simulink library "inp"  (Simulink 8.2)
settings: Solver=ode45  SolverMode=SingleTasking  StartTime=0.0  StopTime=10.0  RelTol=1e-3
undefined symbols (not defined in the file or in its folder): C_TICK_TIME_S, ENUM_FAULTSTATE_FAIL, ENUM_FAULTSTATE_INDETERMINATE, ENUM_FAULTSTATE_PASS, ENUM_KEYSWITCH_ON, FALSE, KINP_ABS1CANCommuOk_flg_ovrdflg, KINP_ABS1CANCommuOk_flg_ovrdval, KINP_ABS1CANPeriodTime_s, KINP_ABS1CANRolCntOk_flg_ovrdflg, KINP_ABS1CANRolCntOk_flg_ovrdval, KINP_ABS2CANChkSumOk_flg_ovrdflg, KINP_ABS2CANChkSumOk_flg_ovrdval, KINP_ABS2CANCommuOk_flg_ovrdflg, KINP_ABS2CANCommuOk_flg_ovrdval, KINP_ABS2CANPeriodTime_s, KINP_ABS2CANRolCntOk_flg_ovrdflg, KINP_ABS2CANRolCntOk_flg_ovrdval, KINP_ABS3CANCommuOk_flg_ovrdflg, KINP_ABS3CANCommuOk_flg_ovrdval, KINP_ABS4CANCommuOk_flg_ovrdflg, KINP_ABS4CANCommuOk_flg_ovrdval, KINP_ABSActive_flg_defval, KINP_ABSActive_flg_invalid, KINP_ABSActive_flg_ovrdflg, KINP_ABSActive_flg_ovrdval, KINP_ABSActive_flg_setvalid, KINP_ABSActive_flg_srvcflg, KINP_ABSActive_flg_srvcval, KINP_ABSCANAlive_flg_ovrdflg, KINP_ABSCANAlive_flg_ovrdval, KINP_ABSCANCommuOk_flg_ovrdflg, KINP_ABSCANCommuOk_flg_ovrdval, KINP_ABSCANValidOk_flg_ovrdflg, KINP_ABSCANValidOk_flg_ovrdval, KINP_ABSFault_flg_defval, KINP_ABSFault_flg_invalid, KINP_ABSFault_flg_ovrdflg, KINP_ABSFault_flg_ovrdval, KINP_ABSFault_flg_setvalid, KINP_ABSFault_flg_srvcflg, KINP_ABSFault_flg_srvcval, KINP_AC1CANCommuOk_flg_ovrdflg, KINP_AC1CANCommuOk_flg_ovrdval, KINP_ACCANAlive_flg_ovrdflg, KINP_ACCANAlive_flg_ovrdval, KINP_ACCANCommuOk_flg_ovrdflg, KINP_ACCANCommuOk_flg_ovrdval, KINP_ACCANValidOk_flg_ovrdflg, KINP_ACCANValidOk_flg_ovrdval, KINP_ACCCancelH_flg_defval, KINP_ACCCancelH_flg_invalid, KINP_ACCCancelH_flg_ovrdflg, KINP_ACCCancelH_flg_ovrdval, KINP_ACCCancelH_flg_setvalid, KINP_ACCCancelH_flg_srvcflg, KINP_ACCCancelH_flg_srvcval, KINP_AccelPedalPosn_pct_defval, KINP_AccelPedalPosn_pct_gain, KINP_AccelPedalPosn_pct_invalid ... (+1866)
libraries: inp x192, hcu_lib x34, simulink x33
(part 4 of 21)

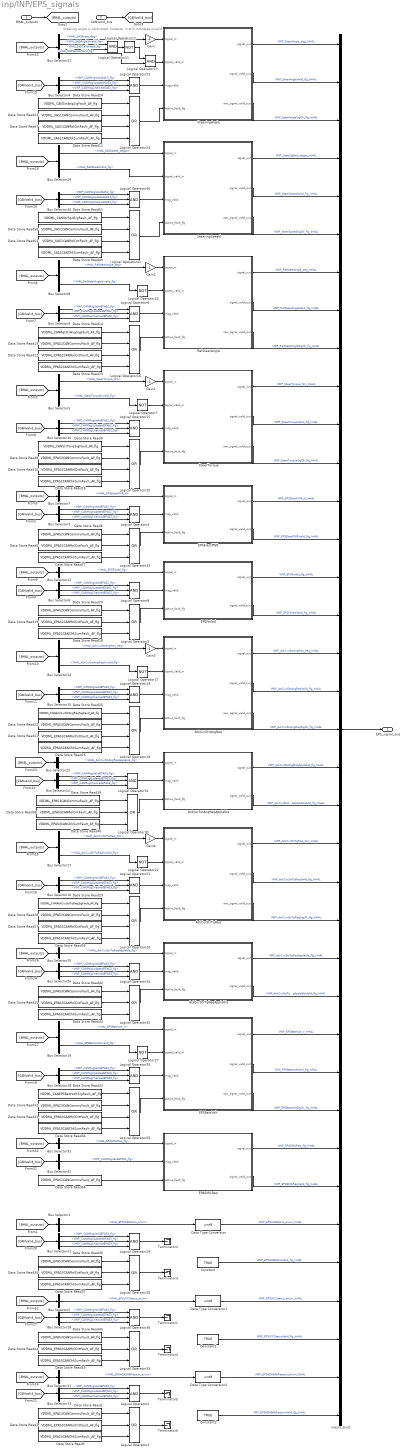
== system inp/INP/EPS_signals ==
  note: Steering angle is calibrated; however, it still indicates invalid.
  BHAL_outputs : Inport  OutDataTypeStr=Bus: BHAL_outputs
  CANvalid_bus : Inport  Port=2
  AckCurStrAngReq : Subsystem
      settings: RTWSystemCode=Inline
  AckCurStrAngReqApplable : Subsystem
      settings: RTWSystemCode=Inline
  AckCurStrTqReq : Subsystem
      settings: RTWSystemCode=Inline
  AckCurStrTqReqApplable : Subsystem
      settings: RTWSystemCode=Inline
  Bus Selector1 : BusSelector  OutputSignals=VHAL_EPSLKAStatus_enum
  Bus Selector{3,6,9..10} (4 alike) : BusSelector  OutputSignals=VINP_CANMsgValidEPAS2_flg,VINP_CANMsgUpdatedEPAS2_flg,VINP_CANMsgCheckedEPAS2_flg
  Bus Selector11 : BusSelector  OutputSignals=VHAL_EPSADASAbReason_enum
  Bus Selector12 : BusSelector  OutputSignals=VHAL_EPSFailed_flg
  Bus Selector{13,15..16,18,21,24,26,28} (8 alike) : BusSelector  OutputSignals=VINP_CANMsgValidEPAS3_flg,VINP_CANMsgUpdatedEPAS3_flg,VINP_CANMsgCheckedEPAS3_flg
  Bus Selector14 : BusSelector  OutputSignals=VHAL_AckCurStrAngReq_deg,VHAL_AckCurStrAngReqInvalid_flg
  Bus Selector17 : BusSelector  OutputSignals=VHAL_AckCurStrTqReq_Nm,VHAL_AckCurStrTqReqInvalid_flg
  Bus Selector19 : BusSelector  OutputSignals=VHAL_EPSBattVolt_V,VHAL_EPSBattVoltInvalid_flg
  Bus Selector2 : BusSelector  OutputSignals=VHAL_EPSLFCStatus_enum
  Bus Selector22 : BusSelector  OutputSignals=VHAL_SASAngle_deg,VHAL_SASAngleInvalid_flg,VHAL_SASCalibrated_flg,VHAL_SASSensorValid_flg
  Bus Selector23 : BusSelector  OutputSignals=VHAL_AckCurStrAngReqApplable_flg
  Bus Selector25 : BusSelector  OutputSignals=VHAL_AckCurStrTqReqApplable_flg
  Bus Selector29 : BusSelector  OutputSignals=VHAL_SASSpeed_degps,VHAL_SASSpeedValid_flg
  Bus Selector30 : BusSelector  OutputSignals=VINP_CANMsgValidSAS1_flg,VINP_CANMsgUpdatedSAS1_flg,VINP_CANMsgCheckedSAS1_flg
  Bus Selector31 : BusSelector  OutputSignals=VHAL_EPASMILReq_flg
  Bus Selector32 : BusSelector  OutputSignals=VINP_CANMsgValidEPAS1_flg
  Bus Selector4 : BusSelector  OutputSignals=VINP_CANMsgValidSAS1_flg,VINP_CANMsgUpdatedSAS1_flg,VINP_CANMsgCheckedSAS1_flg
  Bus Selector5 : BusSelector  OutputSignals=VHAL_SteerTorque_Nm,VHAL_SteerTorqueInvalid_flg
  Bus Selector7 : BusSelector  OutputSignals=VHAL_EPSElectPWR_A
  Bus Selector8 : BusSelector  OutputSignals=VHAL_RelSteerAngle_deg,VHAL_RelSteerAngleInvalid_flg
  Constant : Constant  Value=TRUE
  Constant1 : Constant  Value=TRUE
  Constant2 : Constant  Value=TRUE
  Data Store Read{1,21,27,31,35,38,41,44} (8 alike) : DataStoreRead  DataStoreName=VDDML_EPAS3CANRolCntFault_AF_flg
  Data Store Read{5,10,12,19} (4 alike) : DataStoreRead  DataStoreName=VDDML_EPAS2CANRolCntFault_AF_flg
  Data Store Read11 : DataStoreRead  DataStoreName=VDDML_SAS1CANChkSumFault_AF_flg
  Data Store Read{6,8,13,20} (4 alike) : DataStoreRead  DataStoreName=VDDML_EPAS2CANCommuFault_AF_flg
  Data Store Read14 : DataStoreRead  DataStoreName=VDDML_CANRelStrAngSigFault_AF_flg
  Data Store Read{15..18} (4 alike) : DataStoreRead  DataStoreName=VDDML_EPAS2CANChkSumFault_AF_flg
  Data Store Read{2,22,28,32,36,39,42,45} (8 alike) : DataStoreRead  DataStoreName=VDDML_EPAS3CANCommuFault_AF_flg
  Data Store Read23 : DataStoreRead  DataStoreName=VDDML_SAS1CANCommuFault_AF_flg
  Data Store Read24 : DataStoreRead  DataStoreName=VDDML_CANStrAngSigFault_AF_flg
  Data Store Read25 : DataStoreRead  DataStoreName=VDDML_CANAckCurStrAngReqSigFault_AF_flg
  Data Store Read{3,26,30,34,37,40,43,46} (8 alike) : DataStoreRead  DataStoreName=VDDML_EPAS3CANChkSumFault_AF_flg
  Data Store Read29 : DataStoreRead  DataStoreName=VDDML_CANAckCurStrTqReqSigFault_AF_flg
  Data Store Read33 : DataStoreRead  DataStoreName=VDDML_CANEPSBattVoltSigFault_AF_flg
  Data Store Read49 : DataStoreRead  DataStoreName=VDDML_SAS1CANChkSumFault_AF_flg
  Data Store Read50 : DataStoreRead  DataStoreName=VDDML_SAS1CANCommuFault_AF_flg
  Data Store Read51 : DataStoreRead  DataStoreName=VDDML_CANStrSpdSigFault_AF_flg
  Data Store Read52 : DataStoreRead  DataStoreName=VDDML_SAS1CANRolCntFault_AF_flg
  Data Store Read54 : DataStoreRead  DataStoreName=VDDML_EPAS1CANCommuFault_AF_flg
  Data Store Read7 : DataStoreRead  DataStoreName=VDDML_SAS1CANRolCntFault_AF_flg
  Data Store Read9 : DataStoreRead  DataStoreName=VDDML_CANStrTorqSigFault_AF_flg
  Data Type Conversion : DataTypeConversion  OutDataTypeStr=uint8
  Data Type Conversion1 : DataTypeConversion  OutDataTypeStr=uint8
  Data Type Conversion2 : DataTypeConversion  OutDataTypeStr=uint8
  EPASMILReq : Subsystem
      settings: RTWSystemCode=Inline
  EPSBattVolt : Subsystem
      settings: RTWSystemCode=Inline
  EPSElectPWR : Subsystem
      settings: RTWSystemCode=Inline
  EPSFailed : Subsystem
      settings: RTWSystemCode=Inline
  Gain{,1..4} (5 alike) : Gain  Gain=-1
  Logical Operator{1,3,28,30,32..33,35} (7 alike) : Logic  Tag=struct('inputs','1','comment',struct('text','','placement',1),'logdata',struct('maxnumvals',inf,'plotchannels',[],'stepsize',0,'downsampling',1,'loggingmode',3,'channels',1),'operator','NOT','output',struct('class','default','type','Bool8','description','','address','','unit','','name','$S_$B'),'date',731430.46749816)  Operator=OR  Inputs=3
  Logical Operator{10,16,18,20,24..25,41} (7 alike) : Logic  Tag=struct('inputs','1','comment',struct('text','','placement',1),'logdata',struct('maxnumvals',inf,'plotchannels',[],'stepsize',0,'downsampling',1,'loggingmode',3,'channels',1),'operator','NOT','output',struct('class','default','type','Bool8','description','','address','','unit','','name','$S_$B'),'date',731430.46749816)  Operator=OR  Inputs=4
  Logical Operator11 : Logic  Tag=struct('inputs','1','comment',struct('text','','placement',1),'logdata',struct('maxnumvals',inf,'plotchannels',[],'stepsize',0,'downsampling',1,'loggingmode',3,'channels',1),'operator','NOT','output',struct('class','default','type','Bool8','description','','address','','unit','','name','$S_$B'),'date',731430.46749816)
  Logical Operator{7,12,14,17,22,27} (6 alike) : Logic  Tag=struct('inputs','1','comment',struct('text','','placement',1),'logdata',struct('maxnumvals',inf,'plotchannels',[],'stepsize',0,'downsampling',1,'loggingmode',3,'channels',1),'operator','NOT','output',struct('class','default','type','Bool8','description','','address','','unit','','name','$S_$B'),'date',731430.46749816)  Operator=NOT
  Logical Operator13 : Logic  Tag=struct('inputs','1','comment',struct('text','','placement',1),'logdata',struct('maxnumvals',inf,'plotchannels',[],'stepsize',0,'downsampling',1,'loggingmode',3,'channels',1),'operator','NOT','output',struct('class','default','type','Bool8','description','','address','','unit','','name','$S_$B'),'date',731430.46749816)
  Logical Operator{2,4,6,8,15,19,21,23,26,29,31,34,36,40} (14 alike) : Logic  Tag=struct('inputs','1','comment',struct('text','','placement',1),'logdata',struct('maxnumvals',inf,'plotchannels',[],'stepsize',0,'downsampling',1,'loggingmode',3,'channels',1),'operator','NOT','output',struct('class','default','type','Bool8','description','','address','','unit','','name','$S_$B'),'date',731430.46749816)  Inputs=3
  RelSteerAngle : Subsystem
      settings: RTWSystemCode=Inline
  SteerTorque : Subsystem
      settings: RTWSystemCode=Inline
  SteeringAngle : Subsystem
      settings: RTWSystemCode=Inline
  SteeringSpeed : Subsystem
      settings: RTWSystemCode=Inline
  Terminator{1..6} (6 alike) : Terminator
  inputs_bus2 : BusCreator  Inputs=37
  EPS_signal_bus : Outport
  wires:
  inputs_bus2/1 -> EPS_signal_bus/1
  BHAL_outputs/1 -> [BHAL_outputs]
  EPSElectPWR/1(signal_out) -> inputs_bus2/13  "VINP_EPSElectPWR_A_InHAL"
  Bus Selector7/1 -> EPSElectPWR/1(signal_in)  "<VHAL_EPSElectPWR_A>"
  EPSElectPWR/2(signal_valid_out) -> inputs_bus2/14  "VINP_EPSElectPWRValid_flg_InHAL"
  CANvalid_bus/1 -> [CANvalid_bus]
  Data Store Read6/1 -> Logical Operator33/1
  Data Store Read5/1 -> Logical Operator33/2
  [CANvalid_bus] -> Bus Selector4/1
  SteeringAngle/1(signal_out) -> inputs_bus2/1  "VINP_SteerAngle_deg_InHAL"
  SteeringAngle/2(signal_valid_out) -> inputs_bus2/2  "VINP_SteerAngleValid_flg_InHAL"
  [BHAL_outputs] -> Bus Selector22/1
  Bus Selector22/1 -> Gain/1  "<VHAL_SASAngle_deg>"
  Data Store Read24/1 -> Logical Operator24/1
  Data Store Read23/1 -> Logical Operator24/2
  Logical Operator24/1 -> SteeringAngle/4(Active_fault_flg)
  SteeringAngle/3(raw_signal_valid_out) -> inputs_bus2/3  "VINP_SteerAngleSigOk_flg_InHAL"
  Bus Selector4/1 -> Logical Operator23/1  "<VINP_CANMsgValidSAS1_flg>"
  Bus Selector4/2 -> Logical Operator23/2  "<VINP_CANMsgUpdatedSAS1_flg>"
  Logical Operator23/1 -> SteeringAngle/3(msg_valid)
  Data Store Read7/1 -> Logical Operator24/3
  [BHAL_outputs] -> Bus Selector5/1
  Bus Selector5/1 -> Gain1/1  "<VHAL_SteerTorque_Nm>"
  Bus Selector5/2 -> Logical Operator7/1  "<VHAL_SteerTorqueInvalid_flg>"
  Logical Operator7/1 -> SteerTorque/2(signal_valid_in)
  Data Store Read9/1 -> Logical Operator10/1
  Data Store Read8/1 -> Logical Operator10/2
  Data Store Read10/1 -> Logical Operator10/3
  SteerTorque/1(signal_out) -> inputs_bus2/10  "VINP_SteerTorque_Nm_InHAL"
  SteerTorque/2(signal_valid_out) -> inputs_bus2/11  "VINP_SteerTorqueValid_flg_InHAL"
  SteerTorque/3(raw_signal_valid_out) -> inputs_bus2/12  "VINP_SteerTorqueSigOk_flg_InHAL"
  Logical Operator13/1 -> SteeringAngle/2(signal_valid_in)
  Bus Selector22/2 -> Logical Operator11/1  "<VHAL_SASAngleInvalid_flg>"
  Bus Selector22/3 -> Logical Operator11/2  "<VHAL_SASCalibrated_flg>"
  Logical Operator11/1 -> Logical Operator12/1
  Bus Selector22/4 -> Logical Operator13/2  "<VHAL_SASSensorValid_flg>"
  Logical Operator12/1 -> Logical Operator13/1
  [BHAL_outputs] -> Bus Selector7/1
  Bus Selector4/3 -> Logical Operator23/3  "<VINP_CANMsgCheckedSAS1_flg>"
  Data Store Read11/1 -> Logical Operator24/4
  [BHAL_outputs] -> Bus Selector8/1
  Bus Selector8/1 -> Gain2/1  "<VHAL_RelSteerAngle_deg>"
  Bus Selector8/2 -> Logical Operator14/1  "<VHAL_RelSteerAngleInvalid_flg>"
  Logical Operator14/1 -> RelSteerAngle/2(signal_valid_in)
  Data Store Read14/1 -> Logical Operator16/1
  Data Store Read13/1 -> Logical Operator16/2
  Logical Operator6/1 -> RelSteerAngle/3(msg_valid)
  Data Store Read12/1 -> Logical Operator16/3
  RelSteerAngle/1(signal_out) -> inputs_bus2/7  "VINP_RelSteerAngle_deg_InHAL"
  RelSteerAngle/2(signal_valid_out) -> inputs_bus2/8  "VINP_RelSteerAngleValid_flg_InHAL"
  RelSteerAngle/3(raw_signal_valid_out) -> inputs_bus2/9  "VINP_RelSteerAngleSigOk_flg_InHAL"
  [CANvalid_bus] -> Bus Selector9/1
  Bus Selector9/1 -> Logical Operator6/1  "<VINP_CANMsgValidEPAS2_flg>"
  Bus Selector9/2 -> Logical Operator6/2  "<VINP_CANMsgUpdatedEPAS2_flg>"
  Bus Selector9/3 -> Logical Operator6/3  "<VINP_CANMsgCheckedEPAS2_flg>"
  Logical Operator16/1 -> RelSteerAngle/4(Active_fault_flg)
  Data Store Read15/1 -> Logical Operator16/4
  [CANvalid_bus] -> Bus Selector10/1
  Bus Selector10/1 -> Logical Operator15/1  "<VINP_CANMsgValidEPAS2_flg>"
  Bus Selector10/2 -> Logical Operator15/2  "<VINP_CANMsgUpdatedEPAS2_flg>"
  Bus Selector10/3 -> Logical Operator15/3  "<VINP_CANMsgCheckedEPAS2_flg>"
  Logical Operator15/1 -> SteerTorque/3(msg_valid)
  Logical Operator10/1 -> SteerTorque/4(Active_fault_flg)
  Data Store Read16/1 -> Logical Operator10/4
  [CANvalid_bus] -> Bus Selector3/1
  Bus Selector3/1 -> Logical Operator4/1  "<VINP_CANMsgValidEPAS2_flg>"
  Bus Selector3/2 -> Logical Operator4/2  "<VINP_CANMsgUpdatedEPAS2_flg>"
  Bus Selector3/3 -> Logical Operator4/3  "<VINP_CANMsgCheckedEPAS2_flg>"
  Logical Operator4/1 -> EPSElectPWR/2(msg_valid)
  Logical Operator33/1 -> EPSElectPWR/3(Active_fault_flg)
  Data Store Read17/1 -> Logical Operator33/3
  EPSFailed/1(signal_out) -> inputs_bus2/15  "VINP_EPSFailed_flg_InHAL"
  Bus Selector12/1 -> EPSFailed/1(signal_in)  "<VHAL_EPSFailed_flg>"
  EPSFailed/2(signal_valid_out) -> inputs_bus2/16  "VINP_EPSFailedValid_flg_InHAL"
  Data Store Read20/1 -> Logical Operator3/1
  Data Store Read19/1 -> Logical Operator3/2
  [BHAL_outputs] -> Bus Selector12/1
  [CANvalid_bus] -> Bus Selector6/1
  Bus Selector6/1 -> Logical Operator8/1  "<VINP_CANMsgValidEPAS2_flg>"
  Bus Selector6/2 -> Logical Operator8/2  "<VINP_CANMsgUpdatedEPAS2_flg>"
  Bus Selector6/3 -> Logical Operator8/3  "<VINP_CANMsgCheckedEPAS2_flg>"
  Logical Operator8/1 -> EPSFailed/2(msg_valid)
  Logical Operator3/1 -> EPSFailed/3(Active_fault_flg)
  Data Store Read18/1 -> Logical Operator3/3
  [BHAL_outputs] -> Bus Selector14/1
  Bus Selector14/1 -> Gain3/1  "<VHAL_AckCurStrAngReq_deg>"
  Bus Selector14/2 -> Logical Operator17/1  "<VHAL_AckCurStrAngReqInvalid_flg>"
  Logical Operator17/1 -> AckCurStrAngReq/2(signal_valid_in)
  Data Store Read25/1 -> Logical Operator18/1
  Data Store Read22/1 -> Logical Operator18/2
  Logical Operator19/1 -> AckCurStrAngReq/3(msg_valid)
  Data Store Read21/1 -> Logical Operator18/3
  AckCurStrAngReq/1(signal_out) -> inputs_bus2/17  "VINP_AckCurStrAngReq_deg_InHAL"
  AckCurStrAngReq/2(signal_valid_out) -> inputs_bus2/18  "VINP_AckCurStrAngReqValid_flg_InHAL"
  AckCurStrAngReq/3(raw_signal_valid_out) -> inputs_bus2/19  "VINP_AckCurStrAngReqSigOk_flg_InHAL"
  [CANvalid_bus] -> Bus Selector15/1
  Bus Selector15/1 -> Logical Operator19/1  "<VINP_CANMsgValidEPAS3_flg>"
  Bus Selector15/2 -> Logical Operator19/2  "<VINP_CANMsgUpdatedEPAS3_flg>"
  Bus Selector15/3 -> Logical Operator19/3  "<VINP_CANMsgCheckedEPAS3_flg>"
  Logical Operator18/1 -> AckCurStrAngReq/4(Active_fault_flg)
  Data Store Read26/1 -> Logical Operator18/4
  [BHAL_outputs] -> Bus Selector17/1
  Bus Selector17/1 -> Gain4/1  "<VHAL_AckCurStrTqReq_Nm>"
  Bus Selector17/2 -> Logical Operator22/1  "<VHAL_AckCurStrTqReqInvalid_flg>"
  Logical Operator22/1 -> AckCurStrTqReq/2(signal_valid_in)
  AckCurStrTqReq/1(signal_out) -> inputs_bus2/22  "VINP_AckCurStrTqReq_Nm_InHAL"
  AckCurStrTqReq/2(signal_valid_out) -> inputs_bus2/23  "VINP_AckCurStrTqReqValid_flg_InHAL"
  AckCurStrTqReq/3(raw_signal_valid_out) -> inputs_bus2/24  "VINP_AckCurStrTqReqSigOk_flg_InHAL"
  Data Store Read29/1 -> Logical Operator20/1
  Data Store Read28/1 -> Logical Operator20/2
  Logical Operator21/1 -> AckCurStrTqReq/3(msg_valid)
  Data Store Read27/1 -> Logical Operator20/3
  [CANvalid_bus] -> Bus Selector16/1
  Bus Selector16/1 -> Logical Operator21/1  "<VINP_CANMsgValidEPAS3_flg>"
  Bus Selector16/2 -> Logical Operator21/2  "<VINP_CANMsgUpdatedEPAS3_flg>"
  Bus Selector16/3 -> Logical Operator21/3  "<VINP_CANMsgCheckedEPAS3_flg>"
  Logical Operator20/1 -> AckCurStrTqReq/4(Active_fault_flg)
  Data Store Read30/1 -> Logical Operator20/4
  [BHAL_outputs] -> Bus Selector19/1
  Bus Selector19/1 -> EPSBattVolt/1(signal_in)  "<VHAL_EPSBattVolt_V>"
  Bus Selector19/2 -> Logical Operator27/1  "<VHAL_EPSBattVoltInvalid_flg>"
  Logical Operator27/1 -> EPSBattVolt/2(signal_valid_in)
  EPSBattVolt/1(signal_out) -> inputs_bus2/27  "VINP_EPSBattVolt_V_InHAL"
  EPSBattVolt/2(signal_valid_out) -> inputs_bus2/28  "VINP_EPSBattVoltValid_flg_InHAL"
  EPSBattVolt/3(raw_signal_valid_out) -> inputs_bus2/29  "VINP_EPSBattVoltSigOk_flg_InHAL"
  Data Store Read33/1 -> Logical Operator25/1
  Data Store Read32/1 -> Logical Operator25/2
  Logical Operator26/1 -> EPSBattVolt/3(msg_valid)
  Data Store Read31/1 -> Logical Operator25/3
  [CANvalid_bus] -> Bus Selector18/1
  Bus Selector18/1 -> Logical Operator26/1  "<VINP_CANMsgValidEPAS3_flg>"
  Bus Selector18/2 -> Logical Operator26/2  "<VINP_CANMsgUpdatedEPAS3_flg>"
  Bus Selector18/3 -> Logical Operator26/3  "<VINP_CANMsgCheckedEPAS3_flg>"
  Logical Operator25/1 -> EPSBattVolt/4(Active_fault_flg)
  Data Store Read34/1 -> Logical Operator25/4
  Data Store Read36/1 -> Logical Operator28/1
  Data Store Read35/1 -> Logical Operator28/2
  Logical Operator28/1 -> Terminator3/1
  Data Store Read37/1 -> Logical Operator28/3
  Logical Operator29/1 -> Terminator2/1
  [CANvalid_bus] -> Bus Selector21/1
  Bus Selector21/1 -> Logical Operator29/1  "<VINP_CANMsgValidEPAS3_flg>"
  Bus Selector21/2 -> Logical Operator29/2  "<VINP_CANMsgUpdatedEPAS3_flg>"
  Bus Selector21/3 -> Logical Operator29/3  "<VINP_CANMsgCheckedEPAS3_flg>"
  AckCurStrAngReqApplable/1(signal_out) -> inputs_bus2/20  "VINP_AckCurStrAngReqApplable_flg_InHAL"
  Bus Selector23/1 -> AckCurStrAngReqApplable/1(signal_in)  "<VHAL_AckCurStrAngReqApplable_flg>"
  AckCurStrAngReqApplable/2(signal_valid_out) -> inputs_bus2/21  "VINP_AckCurStrAngReqApplableValid_flg_InHAL"
  Data Store Read39/1 -> Logical Operator30/1
  Data Store Read38/1 -> Logical Operator30/2
  [BHAL_outputs] -> Bus Selector23/1
  Logical Operator30/1 -> AckCurStrAngReqApplable/3(Active_fault_flg)
  Data Store Read40/1 -> Logical Operator30/3
  Logical Operator31/1 -> AckCurStrAngReqApplable/2(msg_valid)
  [CANvalid_bus] -> Bus Selector24/1
  Bus Selector24/1 -> Logical Operator31/1  "<VINP_CANMsgValidEPAS3_flg>"
  Bus Selector24/2 -> Logical Operator31/2  "<VINP_CANMsgUpdatedEPAS3_flg>"
  Bus Selector24/3 -> Logical Operator31/3  "<VINP_CANMsgCheckedEPAS3_flg>"
  AckCurStrTqReqApplable/1(signal_out) -> inputs_bus2/25  "VINP_AckCurStrTqReqApplable_flg_InHAL"
  Bus Selector25/1 -> AckCurStrTqReqApplable/1(signal_in)  "<VHAL_AckCurStrTqReqApplable_flg>"
  AckCurStrTqReqApplable/2(signal_valid_out) -> inputs_bus2/26  "VINP_AckCurStrTqReqApplableValid_flg_InHAL"
  Data Store Read42/1 -> Logical Operator32/1
  Data Store Read41/1 -> Logical Operator32/2
  [BHAL_outputs] -> Bus Selector25/1
  Logical Operator32/1 -> AckCurStrTqReqApplable/3(Active_fault_flg)
  Data Store Read43/1 -> Logical Operator32/3
  Logical Operator34/1 -> AckCurStrTqReqApplable/2(msg_valid)
  [CANvalid_bus] -> Bus Selector26/1
  Bus Selector26/1 -> Logical Operator34/1  "<VINP_CANMsgValidEPAS3_flg>"
  Bus Selector26/2 -> Logical Operator34/2  "<VINP_CANMsgUpdatedEPAS3_flg>"
  Bus Selector26/3 -> Logical Operator34/3  "<VINP_CANMsgCheckedEPAS3_flg>"
  Logical Operator36/1 -> Terminator1/1
  [CANvalid_bus] -> Bus Selector28/1
  Bus Selector28/1 -> Logical Operator36/1  "<VINP_CANMsgValidEPAS3_flg>"
  Bus Selector28/2 -> Logical Operator36/2  "<VINP_CANMsgUpdatedEPAS3_flg>"
  Bus Selector28/3 -> Logical Operator36/3  "<VINP_CANMsgCheckedEPAS3_flg>"
  Data Store Read45/1 -> Logical Operator35/1
  Data Store Read44/1 -> Logical Operator35/2
  Logical Operator35/1 -> Terminator4/1
  Data Store Read46/1 -> Logical Operator35/3
  [CANvalid_bus] -> Bus Selector30/1
  SteeringSpeed/1(signal_out) -> inputs_bus2/4  "VINP_SteerSpeed_degps_InHAL"
  SteeringSpeed/2(signal_valid_out) -> inputs_bus2/5  "VINP_SteerSpeedValid_flg_InHAL"
  Data Store Read51/1 -> Logical Operator41/1
  Data Store Read50/1 -> Logical Operator41/2
  Logical Operator41/1 -> SteeringSpeed/4(Active_fault_flg)
  SteeringSpeed/3(raw_signal_valid_out) -> inputs_bus2/6  "VINP_SteerSpeedSigOk_flg_InHAL"
  Bus Selector30/1 -> Logical Operator40/1  "<VINP_CANMsgValidSAS1_flg>"
  Bus Selector30/2 -> Logical Operator40/2  "<VINP_CANMsgUpdatedSAS1_flg>"
  Logical Operator40/1 -> SteeringSpeed/3(msg_valid)
  Data Store Read52/1 -> Logical Operator41/3
  Bus Selector30/3 -> Logical Operator40/3  "<VINP_CANMsgCheckedSAS1_flg>"
  Data Store Read49/1 -> Logical Operator41/4
  [BHAL_outputs] -> Bus Selector29/1
  Bus Selector29/1 -> SteeringSpeed/1(signal_in)  "<VHAL_SASSpeed_degps>"
  Bus Selector29/2 -> SteeringSpeed/2(signal_valid_in)  "<VHAL_SASSpeedValid_flg>"
  EPASMILReq/1(signal_out) -> inputs_bus2/30  "VINP_EPASMILReq_flg_InHAL"
  Bus Selector31/1 -> EPASMILReq/1(signal_in)  "<VHAL_EPASMILReq_flg>"
  EPASMILReq/2(signal_valid_out) -> inputs_bus2/31  "VINP_EPASMILReqValid_flg_InHAL"
  Data Store Read54/1 -> EPASMILReq/3(Active_fault_flg)
  [BHAL_outputs] -> Bus Selector31/1
  [CANvalid_bus] -> Bus Selector32/1
  Bus Selector32/1 -> EPASMILReq/2(msg_valid)  "<VINP_CANMsgValidEPAS1_flg>"
  Bus Selector1/1 -> Data Type Conversion/1  "<VHAL_EPSLKAStatus_enum>"
  [BHAL_outputs] -> Bus Selector1/1
  Data Type Conversion/1 -> inputs_bus2/32  "VINP_EPSLKAStatus_enum_InHAL"
  Constant/1 -> inputs_bus2/33  "VINP_EPSLKAStatusValid_flg_InHAL"
  Bus Selector2/1 -> Data Type Conversion1/1  "<VHAL_EPSLFCStatus_enum>"
  [BHAL_outputs] -> Bus Selector2/1
  Data Type Conversion1/1 -> inputs_bus2/34  "VINP_EPSLFCStatus_enum_InHAL"
  Constant1/1 -> inputs_bus2/35  "VINP_EPSLFCStatusValid_flg_InHAL"
  Logical Operator2/1 -> Terminator5/1
  [CANvalid_bus] -> Bus Selector13/1
  Bus Selector13/1 -> Logical Operator2/1  "<VINP_CANMsgValidEPAS3_flg>"
  Bus Selector13/2 -> Logical Operator2/2  "<VINP_CANMsgUpdatedEPAS3_flg>"
  Bus Selector13/3 -> Logical Operator2/3  "<VINP_CANMsgCheckedEPAS3_flg>"
  Data Store Read2/1 -> Logical Operator1/1
  Data Store Read1/1 -> Logical Operator1/2
  Logical Operator1/1 -> Terminator6/1
  Data Store Read3/1 -> Logical Operator1/3
  Bus Selector11/1 -> Data Type Conversion2/1  "<VHAL_EPSADASAbReason_enum>"
  [BHAL_outputs] -> Bus Selector11/1
  Data Type Conversion2/1 -> inputs_bus2/36  "VINP_EPSADASAbReason_enum_InHAL"
  Constant2/1 -> inputs_bus2/37  "VINP_EPSADASAbReasonValid_flg_InHAL"
  Gain/1 -> SteeringAngle/1(signal_in)
  Gain2/1 -> RelSteerAngle/1(signal_in)
  Gain1/1 -> SteerTorque/1(signal_in)
  Gain3/1 -> AckCurStrAngReq/1(signal_in)
  Gain4/1 -> AckCurStrTqReq/1(signal_in)

== system inp/INP/HMI_signals ==
  BHAL_outputs : Inport  OutDataTypeStr=Bus: BHAL_outputs
  CANvalid_bus : Inport  Port=2
  ACCCancelH : Subsystem
      settings: RTWSystemCode=Inline
  ACCOnH : Subsystem
      settings: RTWSystemCode=Inline
  ACCResSpdIncH : Subsystem
      settings: RTWSystemCode=Inline
  ACCSetSpdDecH : Subsystem
      settings: RTWSystemCode=Inline
  ACCSpdDecH : Subsystem
      settings: RTWSystemCode=Inline
  ACCSpdIncH : Subsystem
      settings: RTWSystemCode=Inline
  ACCTimeGapDecH : Subsystem
      settings: RTWSystemCode=Inline
  ACCTimeGapIncH : Subsystem
      settings: RTWSystemCode=Inline
  AEBEnableH : Subsystem
      settings: RTWSystemCode=Inline
  Bus Selector{1,3,5,7,9,12,14,16,18..19,22,24,26,28,30,32,34,36,38,40,42..43} (22 alike) : BusSelector  OutputSignals=VINP_CANMsgValidHMI1_flg
  Bus Selector10 : BusSelector  OutputSignals=VHAL_LCSEnableH_flg
  Bus Selector11 : BusSelector  OutputSignals=VHAL_LFCPilotOnH_flg
  Bus Selector13 : BusSelector  OutputSignals=VHAL_LFCPilotCancelH_flg
  Bus Selector15 : BusSelector  OutputSignals=VHAL_ACCOnH_flg
  Bus Selector17 : BusSelector  OutputSignals=VHAL_ACCCancelH_flg
  Bus Selector2 : BusSelector  OutputSignals=VHAL_FCWEnableH_flg
  Bus Selector20 : BusSelector  OutputSignals=VHAL_AEBEnableH_flg
  Bus Selector21 : BusSelector  OutputSignals=VHAL_ACCResSpdIncH_flg
  Bus Selector23 : BusSelector  OutputSignals=VHAL_ACCSetSpdDecH_flg
  Bus Selector25 : BusSelector  OutputSignals=VHAL_ACCSpdIncH_flg
  Bus Selector27 : BusSelector  OutputSignals=VHAL_ACCSpdDecH_flg
  Bus Selector29 : BusSelector  OutputSignals=VHAL_ACCTimeGapIncH_flg
  Bus Selector31 : BusSelector  OutputSignals=VHAL_ACCTimeGapDecH_flg
  Bus Selector33 : BusSelector  OutputSignals=VHAL_IGSAOnH_flg
  Bus Selector35 : BusSelector  OutputSignals=VHAL_IGSAResSpdIncH_flg
  Bus Selector37 : BusSelector  OutputSignals=VHAL_IGSASetSpdDecH_flg
  Bus Selector39 : BusSelector  OutputSignals=VHAL_IGSASwitchModeH_flg
  Bus Selector4 : BusSelector  OutputSignals=VHAL_CSCEnableH_flg
  Bus Selector41 : BusSelector  OutputSignals=VHAL_FCWWarningModeH_enum
  Bus Selector44 : BusSelector  OutputSignals=VHAL_DefMaxACCSetSpeed_kph
  Bus Selector6 : BusSelector  OutputSignals=VHAL_LKAEnableH_flg
  Bus Selector8 : BusSelector  OutputSignals=VHAL_MAPAEnableH_flg
  CSCEnableH : Subsystem
      settings: RTWSystemCode=Inline
  Data Store Read{1..21,40} (22 alike) : DataStoreRead  DataStoreName=VDDML_HMI1CANCommuFault_AF_flg
  DefMaxACCSetSpeed : Subsystem
      settings: RTWSystemCode=Inline
  FCWEnableH : Subsystem
      settings: RTWSystemCode=Inline
  FCWWarningModeH : Subsystem
      settings: RTWSystemCode=Inline
  IGSAOnH : Subsystem
      settings: RTWSystemCode=Inline
  IGSAResSpdIncH : Subsystem
      settings: RTWSystemCode=Inline
  IGSASetSpdDecH : Subsystem
      settings: RTWSystemCode=Inline
  IGSASwitchModeH : Subsystem
      settings: RTWSystemCode=Inline
  LCSEnableH : Subsystem
      settings: RTWSystemCode=Inline
  LFCPilotCancelH : Subsystem
      settings: RTWSystemCode=Inline
  LFCPilotOnH : Subsystem
      settings: RTWSystemCode=Inline
  LKAEnableH : Subsystem
      settings: RTWSystemCode=Inline
  MAPAEnableH : Subsystem
      settings: RTWSystemCode=Inline
  inputs_bus2 : BusCreator  Inputs=44
  HMI_signal_bus : Outport
  wires:
  CANvalid_bus/1 -> [CANvalid_bus]
  BHAL_outputs/1 -> [BHAL_outputs]
  inputs_bus2/1 -> HMI_signal_bus/1
  Bus Selector19/1 -> AEBEnableH/2(msg_valid)  "<VINP_CANMsgValidHMI1_flg>"
  [CANvalid_bus] -> Bus Selector19/1
  Bus Selector20/1 -> AEBEnableH/1(signal_in)  "<VHAL_AEBEnableH_flg>"
  [BHAL_outputs] -> Bus Selector20/1
  AEBEnableH/1(signal_out) -> inputs_bus2/1  "VINP_AEBEnableH_flg_InHAL"
  AEBEnableH/2(signal_valid_out) -> inputs_bus2/2  "VINP_AEBEnableHValid_flg_InHAL"
  Data Store Read40/1 -> AEBEnableH/3(Active_fault_flg)
  Bus Selector1/1 -> FCWEnableH/2(msg_valid)  "<VINP_CANMsgValidHMI1_flg>"
  [CANvalid_bus] -> Bus Selector1/1
  Bus Selector2/1 -> FCWEnableH/1(signal_in)  "<VHAL_FCWEnableH_flg>"
  [BHAL_outputs] -> Bus Selector2/1
  FCWEnableH/1(signal_out) -> inputs_bus2/3  "VINP_FCWEnableH_flg_InHAL"
  FCWEnableH/2(signal_valid_out) -> inputs_bus2/4  "VINP_FCWEnableHValid_flg_InHAL"
  Data Store Read1/1 -> FCWEnableH/3(Active_fault_flg)
  Bus Selector3/1 -> CSCEnableH/2(msg_valid)  "<VINP_CANMsgValidHMI1_flg>"
  [CANvalid_bus] -> Bus Selector3/1
  Bus Selector4/1 -> CSCEnableH/1(signal_in)  "<VHAL_CSCEnableH_flg>"
  [BHAL_outputs] -> Bus Selector4/1
  CSCEnableH/1(signal_out) -> inputs_bus2/5  "VINP_CSCEnableH_flg_InHAL"
  CSCEnableH/2(signal_valid_out) -> inputs_bus2/6  "VINP_CSCEnableHValid_flg_InHAL"
  Data Store Read2/1 -> CSCEnableH/3(Active_fault_flg)
  Bus Selector5/1 -> LKAEnableH/2(msg_valid)  "<VINP_CANMsgValidHMI1_flg>"
  [CANvalid_bus] -> Bus Selector5/1
  Bus Selector6/1 -> LKAEnableH/1(signal_in)  "<VHAL_LKAEnableH_flg>"
  [BHAL_outputs] -> Bus Selector6/1
  LKAEnableH/1(signal_out) -> inputs_bus2/7  "VINP_LKAEnableH_flg_InHAL"
  LKAEnableH/2(signal_valid_out) -> inputs_bus2/8  "VINP_LKAEnableHValid_flg_InHAL"
  Data Store Read3/1 -> LKAEnableH/3(Active_fault_flg)
  Bus Selector7/1 -> MAPAEnableH/2(msg_valid)  "<VINP_CANMsgValidHMI1_flg>"
  [CANvalid_bus] -> Bus Selector7/1
  Bus Selector8/1 -> MAPAEnableH/1(signal_in)  "<VHAL_MAPAEnableH_flg>"
  [BHAL_outputs] -> Bus Selector8/1
  MAPAEnableH/1(signal_out) -> inputs_bus2/9  "VINP_MAPAEnableH_flg_InHAL"
  MAPAEnableH/2(signal_valid_out) -> inputs_bus2/10  "VINP_MAPAEnableHValid_flg_InHAL"
  Data Store Read4/1 -> MAPAEnableH/3(Active_fault_flg)
  Bus Selector9/1 -> LCSEnableH/2(msg_valid)  "<VINP_CANMsgValidHMI1_flg>"
  [CANvalid_bus] -> Bus Selector9/1
  Bus Selector10/1 -> LCSEnableH/1(signal_in)  "<VHAL_LCSEnableH_flg>"
  [BHAL_outputs] -> Bus Selector10/1
  LCSEnableH/1(signal_out) -> inputs_bus2/11  "VINP_LCSEnableH_flg_InHAL"
  LCSEnableH/2(signal_valid_out) -> inputs_bus2/12  "VINP_LCSEnableHValid_flg_InHAL"
  Data Store Read5/1 -> LCSEnableH/3(Active_fault_flg)
  Bus Selector12/1 -> LFCPilotOnH/2(msg_valid)  "<VINP_CANMsgValidHMI1_flg>"
  [CANvalid_bus] -> Bus Selector12/1
  Bus Selector11/1 -> LFCPilotOnH/1(signal_in)  "<VHAL_LFCPilotOnH_flg>"
  [BHAL_outputs] -> Bus Selector11/1
  LFCPilotOnH/1(signal_out) -> inputs_bus2/13  "VINP_LFCPilotOnH_flg_InHAL"
  LFCPilotOnH/2(signal_valid_out) -> inputs_bus2/14  "VINP_LFCPilotOnHValid_flg_InHAL"
  Data Store Read6/1 -> LFCPilotOnH/3(Active_fault_flg)
  Bus Selector14/1 -> LFCPilotCancelH/2(msg_valid)  "<VINP_CANMsgValidHMI1_flg>"
  [CANvalid_bus] -> Bus Selector14/1
  Bus Selector13/1 -> LFCPilotCancelH/1(signal_in)  "<VHAL_LFCPilotCancelH_flg>"
  [BHAL_outputs] -> Bus Selector13/1
  LFCPilotCancelH/1(signal_out) -> inputs_bus2/15  "VINP_LFCPilotCancelH_flg_InHAL"
  LFCPilotCancelH/2(signal_valid_out) -> inputs_bus2/16  "VINP_LFCPilotCancelHValid_flg_InHAL"
  Data Store Read7/1 -> LFCPilotCancelH/3(Active_fault_flg)
  Bus Selector16/1 -> ACCOnH/2(msg_valid)  "<VINP_CANMsgValidHMI1_flg>"
  [CANvalid_bus] -> Bus Selector16/1
  Bus Selector15/1 -> ACCOnH/1(signal_in)  "<VHAL_ACCOnH_flg>"
  [BHAL_outputs] -> Bus Selector15/1
  ACCOnH/1(signal_out) -> inputs_bus2/17  "VINP_ACCOnH_flg_InHAL"
  ACCOnH/2(signal_valid_out) -> inputs_bus2/18  "VINP_ACCOnHValid_flg_InHAL"
  Data Store Read8/1 -> ACCOnH/3(Active_fault_flg)
  Bus Selector18/1 -> ACCCancelH/2(msg_valid)  "<VINP_CANMsgValidHMI1_flg>"
  [CANvalid_bus] -> Bus Selector18/1
  Bus Selector17/1 -> ACCCancelH/1(signal_in)  "<VHAL_ACCCancelH_flg>"
  [BHAL_outputs] -> Bus Selector17/1
  ACCCancelH/1(signal_out) -> inputs_bus2/19  "VINP_ACCCancelH_flg_InHAL"
  ACCCancelH/2(signal_valid_out) -> inputs_bus2/20  "VINP_ACCCancelHValid_flg_InHAL"
  Data Store Read9/1 -> ACCCancelH/3(Active_fault_flg)
  Bus Selector22/1 -> ACCResSpdIncH/2(msg_valid)  "<VINP_CANMsgValidHMI1_flg>"
  [CANvalid_bus] -> Bus Selector22/1
  Bus Selector21/1 -> ACCResSpdIncH/1(signal_in)  "<VHAL_ACCResSpdIncH_flg>"
  [BHAL_outputs] -> Bus Selector21/1
  ACCResSpdIncH/1(signal_out) -> inputs_bus2/21  "VINP_ACCResSpdIncH_flg_InHAL"
  ACCResSpdIncH/2(signal_valid_out) -> inputs_bus2/22  "VINP_ACCResSpdIncHValid_flg_InHAL"
  Data Store Read10/1 -> ACCResSpdIncH/3(Active_fault_flg)
  Bus Selector24/1 -> ACCSetSpdDecH/2(msg_valid)  "<VINP_CANMsgValidHMI1_flg>"
  [CANvalid_bus] -> Bus Selector24/1
  Bus Selector23/1 -> ACCSetSpdDecH/1(signal_in)  "<VHAL_ACCSetSpdDecH_flg>"
  [BHAL_outputs] -> Bus Selector23/1
  ACCSetSpdDecH/1(signal_out) -> inputs_bus2/23  "VINP_ACCSetSpdDecH_flg_InHAL"
  ACCSetSpdDecH/2(signal_valid_out) -> inputs_bus2/24  "VINP_ACCSetSpdDecHValid_flg_InHAL"
  Data Store Read11/1 -> ACCSetSpdDecH/3(Active_fault_flg)
  Bus Selector26/1 -> ACCSpdIncH/2(msg_valid)  "<VINP_CANMsgValidHMI1_flg>"
  [CANvalid_bus] -> Bus Selector26/1
  Bus Selector25/1 -> ACCSpdIncH/1(signal_in)  "<VHAL_ACCSpdIncH_flg>"
  [BHAL_outputs] -> Bus Selector25/1
  ACCSpdIncH/1(signal_out) -> inputs_bus2/25  "VINP_ACCSpdIncH_flg_InHAL"
  ACCSpdIncH/2(signal_valid_out) -> inputs_bus2/26  "VINP_ACCSpdIncHValid_flg_InHAL"
  Data Store Read12/1 -> ACCSpdIncH/3(Active_fault_flg)
  Bus Selector28/1 -> ACCSpdDecH/2(msg_valid)  "<VINP_CANMsgValidHMI1_flg>"
  [CANvalid_bus] -> Bus Selector28/1
  Bus Selector27/1 -> ACCSpdDecH/1(signal_in)  "<VHAL_ACCSpdDecH_flg>"
  [BHAL_outputs] -> Bus Selector27/1
  ACCSpdDecH/1(signal_out) -> inputs_bus2/27  "VINP_ACCSpdDecH_flg_InHAL"
  ACCSpdDecH/2(signal_valid_out) -> inputs_bus2/28  "VINP_ACCSpdDecHValid_flg_InHAL"
  Data Store Read13/1 -> ACCSpdDecH/3(Active_fault_flg)
  Bus Selector30/1 -> ACCTimeGapIncH/2(msg_valid)  "<VINP_CANMsgValidHMI1_flg>"
  [CANvalid_bus] -> Bus Selector30/1
  Bus Selector29/1 -> ACCTimeGapIncH/1(signal_in)  "<VHAL_ACCTimeGapIncH_flg>"
  [BHAL_outputs] -> Bus Selector29/1
  ACCTimeGapIncH/1(signal_out) -> inputs_bus2/29  "VINP_ACCTimeGapIncH_flg_InHAL"
  ACCTimeGapIncH/2(signal_valid_out) -> inputs_bus2/30  "VINP_ACCTimeGapIncHValid_flg_InHAL"
  Data Store Read14/1 -> ACCTimeGapIncH/3(Active_fault_flg)
  Bus Selector32/1 -> ACCTimeGapDecH/2(msg_valid)  "<VINP_CANMsgValidHMI1_flg>"
  [CANvalid_bus] -> Bus Selector32/1
  Bus Selector31/1 -> ACCTimeGapDecH/1(signal_in)  "<VHAL_ACCTimeGapDecH_flg>"
  [BHAL_outputs] -> Bus Selector31/1
  ACCTimeGapDecH/1(signal_out) -> inputs_bus2/31  "VINP_ACCTimeGapDecH_flg_InHAL"
  ACCTimeGapDecH/2(signal_valid_out) -> inputs_bus2/32  "VINP_ACCTimeGapDecHValid_flg_InHAL"
  Data Store Read15/1 -> ACCTimeGapDecH/3(Active_fault_flg)
  Bus Selector34/1 -> IGSAOnH/2(msg_valid)  "<VINP_CANMsgValidHMI1_flg>"
  [CANvalid_bus] -> Bus Selector34/1
  Bus Selector33/1 -> IGSAOnH/1(signal_in)  "<VHAL_IGSAOnH_flg>"
  [BHAL_outputs] -> Bus Selector33/1
  IGSAOnH/1(signal_out) -> inputs_bus2/33  "VINP_IGSAOnH_flg_InHAL"
  IGSAOnH/2(signal_valid_out) -> inputs_bus2/34  "VINP_IGSAOnHValid_flg_InHAL"
  Data Store Read16/1 -> IGSAOnH/3(Active_fault_flg)
  Bus Selector36/1 -> IGSAResSpdIncH/2(msg_valid)  "<VINP_CANMsgValidHMI1_flg>"
  [CANvalid_bus] -> Bus Selector36/1
  Bus Selector35/1 -> IGSAResSpdIncH/1(signal_in)  "<VHAL_IGSAResSpdIncH_flg>"
  [BHAL_outputs] -> Bus Selector35/1
  IGSAResSpdIncH/1(signal_out) -> inputs_bus2/35  "VINP_IGSAResSpdIncH_flg_InHAL"
  IGSAResSpdIncH/2(signal_valid_out) -> inputs_bus2/36  "VINP_IGSAResSpdIncHValid_flg_InHAL"
  Data Store Read17/1 -> IGSAResSpdIncH/3(Active_fault_flg)
  Bus Selector38/1 -> IGSASetSpdDecH/2(msg_valid)  "<VINP_CANMsgValidHMI1_flg>"
  [CANvalid_bus] -> Bus Selector38/1
  Bus Selector37/1 -> IGSASetSpdDecH/1(signal_in)  "<VHAL_IGSASetSpdDecH_flg>"
  [BHAL_outputs] -> Bus Selector37/1
  IGSASetSpdDecH/1(signal_out) -> inputs_bus2/37  "VINP_IGSASetSpdDecH_flg_InHAL"
  IGSASetSpdDecH/2(signal_valid_out) -> inputs_bus2/38  "VINP_IGSASetSpdDecHValid_flg_InHAL"
  Data Store Read18/1 -> IGSASetSpdDecH/3(Active_fault_flg)
  Bus Selector40/1 -> IGSASwitchModeH/2(msg_valid)  "<VINP_CANMsgValidHMI1_flg>"
  [CANvalid_bus] -> Bus Selector40/1
  Bus Selector39/1 -> IGSASwitchModeH/1(signal_in)  "<VHAL_IGSASwitchModeH_flg>"
  [BHAL_outputs] -> Bus Selector39/1
  IGSASwitchModeH/1(signal_out) -> inputs_bus2/39  "VINP_IGSASwitchModeH_flg_InHAL"
  IGSASwitchModeH/2(signal_valid_out) -> inputs_bus2/40  "VINP_IGSASwitchModeHValid_flg_InHAL"
  Data Store Read19/1 -> IGSASwitchModeH/3(Active_fault_flg)
  Bus Selector42/1 -> FCWWarningModeH/2(msg_valid)  "<VINP_CANMsgValidHMI1_flg>"
  [CANvalid_bus] -> Bus Selector42/1
  Bus Selector41/1 -> FCWWarningModeH/1(signal_in)  "<VHAL_FCWWarningModeH_enum>"
  [BHAL_outputs] -> Bus Selector41/1
  FCWWarningModeH/1(signal_out) -> inputs_bus2/41  "VINP_FCWWarningModeH_enum_InHAL"
  FCWWarningModeH/2(signal_valid_out) -> inputs_bus2/42  "VINP_FCWWarningModeHValid_flg_InHAL"
  Data Store Read20/1 -> FCWWarningModeH/3(Active_fault_flg)
  Bus Selector43/1 -> DefMaxACCSetSpeed/2(msg_valid)  "<VINP_CANMsgValidHMI1_flg>"
  [CANvalid_bus] -> Bus Selector43/1
  DefMaxACCSetSpeed/1(signal_out) -> inputs_bus2/43  "VINP_DefMaxACCSetSpeed_kph_InHAL"
  [BHAL_outputs] -> Bus Selector44/1
  Bus Selector44/1 -> DefMaxACCSetSpeed/1(signal_in)  "<VHAL_DefMaxACCSetSpeed_kph>"
  DefMaxACCSetSpeed/2(signal_valid_out) -> inputs_bus2/44  "VINP_DefMaxACCSetSpeedValid_flg_InHAL"
  Data Store Read21/1 -> DefMaxACCSetSpeed/3(Active_fault_flg)

== system inp/INP/HWIO_signals ==
  BHAL_outputs : Inport  OutDataTypeStr=Bus: BHAL_outputs
  Task_Time_s : Inport  Port=2
  BatteryVolt : Subsystem
  Bus Selector1 : BusSelector  OutputSignals=VHAL_VREF1Fault_enum,VHAL_VREF2Fault_enum
  Bus Selector15 : BusSelector  OutputSignals=VHAL_VREF1Fault_enum,VHAL_VREF2Fault_enum
  Bus Selector2 : BusSelector  OutputSignals=VHAL_VbatprotVolt_V
  Bus Selector3 : BusSelector  OutputSignals=VHAL_KeySwitchStatus_enum
  Bus Selector5 : BusSelector  OutputSignals=VHAL_IgnitionVolt_V
  Data Store Read1 : DataStoreRead  DataStoreName=VDDML_VREF1Fault_AF_flg
  Data Store Read10 : DataStoreRead  DataStoreName=VDDML_ADCFault_AF_flg
  Data Store Read2 : DataStoreRead  DataStoreName=VDDML_IgnVoltRangHighFault_AF_flg
  Data Store Read3 : DataStoreRead  DataStoreName=VDDML_IgnVoltRangLowFault_AF_flg
  Data Store Read4 : DataStoreRead  DataStoreName=VDDML_VREF2Fault_AF_flg
  Data Store Read5 : DataStoreRead  DataStoreName=VDDML_VREF1Fault_AF_flg
  Data Store Read6 : DataStoreRead  DataStoreName=VDDML_BattVoltRangHighFault_AF_flg
  Data Store Read7 : DataStoreRead  DataStoreName=VDDML_BattVoltRangLowFault_AF_flg
  Data Store Read8 : DataStoreRead  DataStoreName=VDDML_VREF2Fault_AF_flg
  Data Store Read9 : DataStoreRead  DataStoreName=VDDML_ADCFault_AF_flg
  IgnitionVolt : Subsystem
  Logical Operator1 : Logic  Operator=OR  Inputs=5  AllPortsSameDT=off  OutDataTypeStr=boolean
  Logical Operator2 : Logic  Operator=OR  Inputs=5  AllPortsSameDT=off  OutDataTypeStr=boolean
  MinMax : MinMax  Function=max  Inputs=2
  MinMax1 : MinMax  Function=max  Inputs=2
  VHAL_ADCFault_enum : Constant  Value=0  OutDataTypeStr=uint8
  VHAL_ADCFault_enum1 : Constant  Value=0  OutDataTypeStr=uint8
  inputs_bus1 : BusCreator  Inputs=8
  HWIO_signal_bus : Outport
  wires:
  Task_Time_s/1 -> [Task_Time_s]
  BHAL_outputs/1 -> [BHAL_outputs]
  inputs_bus1/1 -> HWIO_signal_bus/1
  Bus Selector15/1 -> MinMax/1  "<VHAL_VREF1Fault_enum>"
  [BHAL_outputs] -> Bus Selector15/1
  [Task_Time_s] -> IgnitionVolt/5(sample_time)
  IgnitionVolt/4(signal_valid) -> inputs_bus1/4  "VINP_IgnitionVoltValid_flg_InHAL"
  IgnitionVolt/2(fault_lo) -> inputs_bus1/2  "VINP_IgnitionVoltFltRangeLow_enum_InHAL"
  IgnitionVolt/1(fault_hi) -> inputs_bus1/1  "VINP_IgnitionVoltFltRangeHigh_enum_InHAL"
  IgnitionVolt/3(signal_out) -> inputs_bus1/3  "VINP_IgnitionVolt_V_InHAL"
  Bus Selector5/1 -> IgnitionVolt/1(signal_raw)  "<VHAL_IgnitionVolt_V>"
  [BHAL_outputs] -> Bus Selector5/1
  VHAL_ADCFault_enum/1 -> IgnitionVolt/3(adc_fault)
  Data Store Read9/1 -> Logical Operator1/1
  Logical Operator1/1 -> IgnitionVolt/6(Active_fault_flg)
  Data Store Read1/1 -> Logical Operator1/2
  Data Store Read2/1 -> Logical Operator1/4
  Data Store Read3/1 -> Logical Operator1/5
  MinMax/1 -> IgnitionVolt/4(vref_fault)
  Bus Selector15/2 -> MinMax/2  "<VHAL_VREF2Fault_enum>"
  Data Store Read4/1 -> Logical Operator1/3
  Bus Selector1/1 -> MinMax1/1  "<VHAL_VREF1Fault_enum>"
  [BHAL_outputs] -> Bus Selector1/1
  [Task_Time_s] -> BatteryVolt/4(sample_time)
  Bus Selector2/1 -> BatteryVolt/1(signal_raw)  "<VHAL_VbatprotVolt_V>"
  [BHAL_outputs] -> Bus Selector2/1
  VHAL_ADCFault_enum1/1 -> BatteryVolt/2(adc_fault)
  Data Store Read10/1 -> Logical Operator2/1
  Logical Operator2/1 -> BatteryVolt/5(Active_fault_flg)
  Data Store Read5/1 -> Logical Operator2/2
  Data Store Read6/1 -> Logical Operator2/4
  Data Store Read7/1 -> Logical Operator2/5
  MinMax1/1 -> BatteryVolt/3(vref_fault)
  Bus Selector1/2 -> MinMax1/2  "<VHAL_VREF2Fault_enum>"
  Data Store Read8/1 -> Logical Operator2/3
  BatteryVolt/1(fault_hi) -> inputs_bus1/5  "VINP_BatteryVoltFltRangeHigh_enum_InHAL"
  BatteryVolt/2(fault_lo) -> inputs_bus1/6  "VINP_BatteryVoltFltRangeLow_enum_InHAL"
  BatteryVolt/3(signal_out) -> inputs_bus1/7  "VINP_BatteryVolt_V_InHAL"
  BatteryVolt/4(signal_valid) -> inputs_bus1/8  "VINP_BatteryVoltValid_flg_InHAL"
  [BHAL_outputs] -> Bus Selector3/1
  Bus Selector3/1 -> IgnitionVolt/2(VHAL_KeySwitchStatus_enum)  "<VHAL_KeySwitchStatus_enum>"

== system inp/INP/InputSignals ==
  ECM_signal_bus : Inport
  TCM_signal_bus : Inport  Port=2
  BCM_signal_bus : Inport  Port=3
  ABS_signal_bus : Inport  Port=4
  EPS_signal_bus : Inport  Port=5
  METER_signal_bus : Inport  Port=6
  AC_signal_bus : Inport  Port=7
  EPB_signal_bus : Inport  Port=8
  CSW_signal_bus : Inport  Port=9
  VCU_signal_bus : Inport  Port=10
  HMI_signal_bus : Inport  Port=11
  EPM_signal_bus : Inport  Port=12
  HWIO_signal_bus : Inport  Port=13
  LLSW_signal_bus : Inport  Port=14
  Task_Time_s : Inport  Port=15
  ProtCC_bus : Inport  Port=16
  CANvalid_bus : Inport  Port=17
  Diagnosis : Subsystem
  DirectPassThrough : Subsystem
  SignalProcess : Subsystem
  BINP_outputs : Outport
  wires:
  DirectPassThrough/1(BINP_outputs) -> BINP_outputs/1
  Task_Time_s/1 -> SignalProcess/15(Task_Time_s)
  SignalProcess/1(INP_calc_bus) -> DirectPassThrough/15(INP_calc_bus)
  ECM_signal_bus/1 -> Diagnosis/1(ECM_signal_bus), SignalProcess/1(ECM_signal_bus), DirectPassThrough/1(ECM_signal_bus)
  TCM_signal_bus/1 -> Diagnosis/2(TCM_signal_bus), SignalProcess/2(TCM_signal_bus), DirectPassThrough/2(TCM_signal_bus)
  BCM_signal_bus/1 -> Diagnosis/3(BCM_signal_bus), SignalProcess/3(BCM_signal_bus), DirectPassThrough/3(BCM_signal_bus)
  ABS_signal_bus/1 -> Diagnosis/4(ABS_signal_bus), SignalProcess/4(ABS_signal_bus), DirectPassThrough/4(ABS_signal_bus)
  EPS_signal_bus/1 -> Diagnosis/5(EPS_signal_bus), SignalProcess/5(EPS_signal_bus), DirectPassThrough/5(EPS_signal_bus)
  EPM_signal_bus/1 -> DirectPassThrough/12(EPM_signal_bus), SignalProcess/12(EPM_signal_bus), Diagnosis/12(EPM_signal_bus)
  HWIO_signal_bus/1 -> DirectPassThrough/13(HWIO_signal_bus), SignalProcess/13(HWIO_signal_bus), Diagnosis/13(HWIO_signal_bus)
  LLSW_signal_bus/1 -> DirectPassThrough/14(LLSW_signal_bus), SignalProcess/14(LLSW_signal_bus), Diagnosis/14(LLSW_signal_bus)
  ProtCC_bus/1 -> Diagnosis/15(ProtCC_bus)
  CANvalid_bus/1 -> Diagnosis/16(CANvalid_bus)
  Diagnosis/1(INP_diag_bus) -> DirectPassThrough/16(INP_diag_bus)
  METER_signal_bus/1 -> Diagnosis/6(METER_signal_bus), SignalProcess/6(METER_signal_bus), DirectPassThrough/6(METER_signal_bus)
  AC_signal_bus/1 -> Diagnosis/7(AC_signal_bus), SignalProcess/7(AC_signal_bus), DirectPassThrough/7(AC_signal_bus)
  EPB_signal_bus/1 -> Diagnosis/8(EPB_signal_bus), SignalProcess/8(EPB_signal_bus), DirectPassThrough/8(EPB_signal_bus)
  VCU_signal_bus/1 -> DirectPassThrough/10(VCU_signal_bus), SignalProcess/10(VCU_signal_bus), Diagnosis/10(VCU_signal_bus)
  HMI_signal_bus/1 -> DirectPassThrough/11(HMI_signal_bus), SignalProcess/11(HMI_signal_bus), Diagnosis/11(HMI_signal_bus)
  CSW_signal_bus/1 -> DirectPassThrough/9(CSW_signal_bus), Diagnosis/9(CSW_signal_bus), SignalProcess/9(CSW_signal_bus)

== system inp/INP/LLSW_signals ==
  BHAL_outputs : Inport  OutDataTypeStr=Bus: BHAL_outputs
  Bus Selector19 : BusSelector  OutputSignals=VHAL_AppTime_ms,VHAL_ADCFault_enum,VHAL_EEPROMFault_enum,VHAL_RAMFault_enum,VHAL_ROMFault_enum,VHAL_VREF1Fault_enum,VHAL_VREF2Fault_enum
  inputs_bus2 : BusCreator  Inputs=7
  LLSW_signal_bus : Outport
  wires:
  inputs_bus2/1 -> LLSW_signal_bus/1
  BHAL_outputs/1 -> Bus Selector19/1
  Bus Selector19/1 -> inputs_bus2/1  "<VHAL_AppTime_ms>"
  Bus Selector19/3 -> inputs_bus2/3  "<VHAL_EEPROMFault_enum>"
  Bus Selector19/4 -> inputs_bus2/4  "<VHAL_RAMFault_enum>"
  Bus Selector19/5 -> inputs_bus2/5  "<VHAL_ROMFault_enum>"
  Bus Selector19/6 -> inputs_bus2/6  "<VHAL_VREF1Fault_enum>"
  Bus Selector19/7 -> inputs_bus2/7  "<VHAL_VREF2Fault_enum>"
  Bus Selector19/2 -> inputs_bus2/2  "<VHAL_ADCFault_enum>"
